simulink model "ClimaRateLimMgr"  (R2022b)
settings: Solver=FixedStepDiscrete  StartTime=0.0  StopTime=10.0  FixedStep=0.2  RelTol=1e-3
(part 2 of 2)

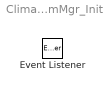
== system ClimaRateLimMgr/ClimaRateLimMgr_Init ==
  Event Listener : EventListener  EventName=reset

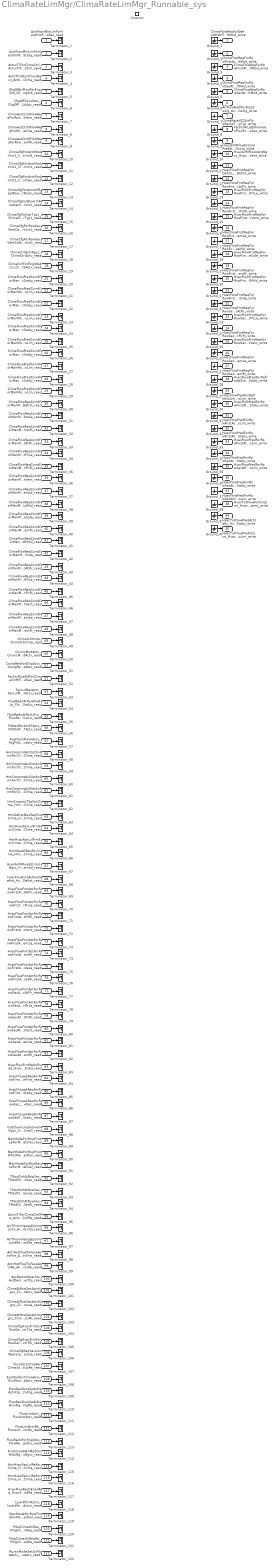
== system ClimaRateLimMgr/ClimaRateLimMgr_Runnable_sys ==
  ActrPosnMinLimForVentForRowSec_ActrPosnMinLimForVentForRowSec_read : Inport
  ActrPosnMinLimForVentForRowSecPredForStaby_ActrPosnMinLimForVentForRowSecPredForStaby_read : Inport  Port=2
  ActvnTiForClimaCtrl_ActvnTiForClimaCtrl_read : Inport  Port=3
  AmbTFildForClimaNgcc_AmbTFildForClima_read : Inport  Port=4
  ChgOfAirFlowForEngStb_ChgOfAirFlowForEngStb_read : Inport  Port=5
  ChgOfFanLvlAut_ChgOfFanLvlAut_read : Inport  Port=6
  ClimaAutCtrldFlowReqForRowSecAtVent_ClimaAutCtrldFlowReqForRowSecAtVent_read : Inport  Port=7
  ClimaAutCtrldFlowReqForRowSecAtVent_ClimaAutCtrldFlowReqForRowSecAtVentLe_read : Inport  Port=8
  ClimaAutCtrldFlowReqForRowSecAtVent_ClimaAutCtrldFlowReqForRowSecAtVentRi_read : Inport  Port=9
  ClimaCfgForActrModVrnt1_ClimaCfgForActrInd1_read : Inport  Port=10
  ClimaCfgForActrModVrnt1_ClimaCfgForActrModVrnt1_read : Inport  Port=11
  ClimaCfgForActrModVrnt1_ClimaCfgForActrModVrntCen_read : Inport  Port=12
  ClimaCfgForAmntOfSeatRow1_ClimaCfgForAmntOfSeatRow1_read : Inport  Port=13
  ClimaCfgForBlowrInRowSecVentSidePrsnt1_ClimaCfgForBlowrInRowSecVentSidePrsnt1_read : Inport  Port=14
  ClimaCfgForCarTyp1_ClimaCfgForCarTyp1_read : Inport  Port=15
  ClimaCfgForRowSecVentCentrVrnt1_ClimaCfgForRowSecVentCentrVrnt1_read : Inport  Port=16
  ClimaCfgForRowSecVentSidePrsnt1_ClimaCfgForRowSecVentSidePrsnt1_read : Inport  Port=17
  ClimaCtrlActvNgcc_ClimaCtrlActv_read : Inport  Port=18
  ClimaCtrlForEngStbActv_ClimaCtrlForEngStbActv_read : Inport  Port=19
  ClimaFlowPredCorrdForManModForDefrstAtStaby_ClimaFlowPredCorrdForManModForDefrstAtStaby_read : Inport  Port=20
  ClimaFlowPredCorrdForManModForDefrstNoLim_ClimaFlowPredCorrdForManModForDefrstNoLim_read : Inport  Port=21
  ClimaFlowPredCorrdForManModForRowFirstAtFlrAtStaby_ClimaFlowPredCorrdForManModForRowFirstAtFlrAtStaby_read : Inport  Port=22
  ClimaFlowPredCorrdForManModForRowFirstAtFlrNoLim_ClimaFlowPredCorrdForManModForRowFirstAtFlrNoLim_read : Inport  Port=23
  ClimaFlowPredCorrdForManModForRowFirstAtVentAtStaby_ClimaFlowPredCorrdForManModForRowFirstAtVentAtStaby_read : Inport  Port=24
  ClimaFlowPredCorrdForManModForRowFirstAtVentNoLim_ClimaFlowPredCorrdForManModForRowFirstAtVentNoLim_read : Inport  Port=25
  ClimaFlowPredCorrdForManModForRowSecAtFlrAtStaby_ClimaFlowPredCorrdForManModForRowSecAtFlrAtStaby_read : Inport  Port=26
  ClimaFlowPredCorrdForManModForRowSecAtFlrNoLim_ClimaFlowPredCorrdForManModForRowSecAtFlrNoLim_read : Inport  Port=27
  ClimaFlowPredCorrdForManModForRowSecAtVentAtStaby_ClimaFlowPredCorrdForManModForRowSecAtVentAtStaby_read : Inport  Port=28
  ClimaFlowPredCorrdForManModForRowSecAtVentNoLim_ClimaFlowPredCorrdForManModForRowSecAtVentNoLim_read : Inport  Port=29
  ClimaFlowReqCorrdForManModForDefrst_ClimaFlowReqCorrdForManModForDefrst_read : Inport  Port=30
  ClimaFlowReqCorrdForManModForDefrst_ClimaFlowReqCorrdForManModForDefrstLe_read : Inport  Port=31
  ClimaFlowReqCorrdForManModForDefrst_ClimaFlowReqCorrdForManModForDefrstRi_read : Inport  Port=32
  ClimaFlowReqCorrdForManModForRowFirstAtFlr_ClimaFlowReqCorrdForManModForRowFirstAtFlr_read : Inport  Port=33
  ClimaFlowReqCorrdForManModForRowFirstAtFlr_ClimaFlowReqCorrdForManModForRowFirstAtFlrLe_read : Inport  Port=34
  ClimaFlowReqCorrdForManModForRowFirstAtFlr_ClimaFlowReqCorrdForManModForRowFirstAtFlrRi_read : Inport  Port=35
  ClimaFlowReqCorrdForManModForRowFirstAtVent_ClimaFlowReqCorrdForManModForRowFirstAtVent_read : Inport  Port=36
  ClimaFlowReqCorrdForManModForRowFirstAtVent_ClimaFlowReqCorrdForManModForRowFirstAtVentLe_read : Inport  Port=37
  ClimaFlowReqCorrdForManModForRowFirstAtVent_ClimaFlowReqCorrdForManModForRowFirstAtVentLeMid_read : Inport  Port=38
  ClimaFlowReqCorrdForManModForRowFirstAtVent_ClimaFlowReqCorrdForManModForRowFirstAtVentLeSide_read : Inport  Port=39
  ClimaFlowReqCorrdForManModForRowFirstAtVent_ClimaFlowReqCorrdForManModForRowFirstAtVentRi_read : Inport  Port=40
  ClimaFlowReqCorrdForManModForRowFirstAtVent_ClimaFlowReqCorrdForManModForRowFirstAtVentRiMid_read : Inport  Port=41
  ClimaFlowReqCorrdForManModForRowFirstAtVent_ClimaFlowReqCorrdForManModForRowFirstAtVentRiSide_read : Inport  Port=42
  ClimaFlowReqCorrdForManModForRowSecAtFlr_ClimaFlowReqCorrdForManModForRowSecAtFlr_read : Inport  Port=43
  ClimaFlowReqCorrdForManModForRowSecAtFlr_ClimaFlowReqCorrdForManModForRowSecAtFlrLe_read : Inport  Port=44
  ClimaFlowReqCorrdForManModForRowSecAtFlr_ClimaFlowReqCorrdForManModForRowSecAtFlrRi_read : Inport  Port=45
  ClimaFlowReqCorrdForManModForRowSecAtVent_ClimaFlowReqCorrdForManModForRowSecAtVent_read : Inport  Port=46
  ClimaFlowReqCorrdForManModForRowSecAtVent_ClimaFlowReqCorrdForManModForRowSecAtVentLe_read : Inport  Port=47
  ClimaFlowReqCorrdForManModForRowSecAtVent_ClimaFlowReqCorrdForManModForRowSecAtVentRi_read : Inport  Port=48
  ClimaStStrtUp_ClimaStStrtUp_read : Inport  Port=49
  CnvincModActv_CnvincModActv_read : Inport  Port=50
  CoolgReqForDispActv_CoolgReqForDispActv_read : Inport  Port=51
  FacForRiLeDifForClimaCmftFlowForRowSec_FacForRiLeDifForClimaCmftFlowForRowSec_read : Inport  Port=52
  FanLvlMaxActv_FanLvlMaxActv_read : Inport  Port=53
  FlowRednAtPwrAllwdLo_FlowRednAtPwrAllwdLo_read : Inport  Port=54
  FlowRednAtPwrLvlLo_FlowRednAtPwrLvlLo_read : Inport  Port=55
  FltModForAmbTActv_FltModForAmbTActv_read : Inport  Port=56
  FogFlashPrevnActv_FogFlashPrevnActv_read : Inport  Port=57
  HmiCmptmtAirDistbnFrntForClima_HmiCmptmtAirDistbnFrntForClima_read : Inport  Port=58
  HmiCmptmtAirDistbnFrntForClima_HmiCmptmtAirDistbnFrntRiForClima_read : Inport  Port=59
  HmiCmptmtAirDistbnFrntForClima_HmiCmptmtAirDistbnReForClima_read : Inport  Port=60
  HmiCmptmtAirDistbnFrntForClima_HmiCmptmtAirDistbnReRiForClima_read : Inport  Port=61
  HmiCmptmtTSpForClima_HmiCmptmtTSpForClima_read : Inport  Port=62
  HmiDefrstMaxReqForClima_HmiDefrstMaxReqForClima_read : Inport  Port=63
  HmiHvacFanLvlFrntForClima_HmiHvacFanLvlFrntForClima_read : Inport  Port=64
  HmiHvacFanLvlFrntForClima_HmiHvacFanLvlFrntRiForClima_read : Inport  Port=65
  HmiMaxACReqForClima_HmiMaxACReqForClima_read : Inport  Port=66
  HvacAirMFlowEstimd_Ngcc_HvacAirMFlowEstimd_read : Inport  Port=67
  HvacFlowFrntActForDefrst_HvacFlowFrntActForDefrst_read : Inport  Port=68
  HvacFlowFrntActForRowFirstAtFlr_HvacFlowFrntActForRowFirstAtFlr_read : Inport  Port=69
  HvacFlowFrntActForRowFirstAtFlr_HvacFlowFrntActForRowFirstAtFlrLe_read : Inport  Port=70
  HvacFlowFrntActForRowFirstAtFlr_HvacFlowFrntActForRowFirstAtFlrRi_read : Inport  Port=71
  HvacFlowFrntActForRowFirstAtVent_HvacFlowFrntActForRowFirstAtVent_read : Inport  Port=72
  HvacFlowFrntActForRowFirstAtVent_HvacFlowFrntActForRowFirstAtVentLe_read : Inport  Port=73
  HvacFlowFrntActForRowFirstAtVent_HvacFlowFrntActForRowFirstAtVentRi_read : Inport  Port=74
  HvacFlowFrntActForRowFirstAtVent_HvacFlowFrntActForRowFirstAtVentSideLe_read : Inport  Port=75
  HvacFlowFrntActForRowFirstAtVent_HvacFlowFrntActForRowFirstAtVentSideRi_read : Inport  Port=76
  HvacFlowFrntActForRowSecAtFlr_HvacFlowFrntActForRowSecAtFlr_read : Inport  Port=77
  HvacFlowFrntActForRowSecAtFlr_HvacFlowFrntActForRowSecAtFlrLe_read : Inport  Port=78
  HvacFlowFrntActForRowSecAtFlr_HvacFlowFrntActForRowSecAtFlrRi_read : Inport  Port=79
  HvacFlowFrntActForRowSecAtVent_HvacFlowFrntActForRowSecAtVent_read : Inport  Port=80
  HvacFlowFrntActForRowSecAtVent_HvacFlowFrntActForRowSecAtVentLe_read : Inport  Port=81
  HvacFlowFrntActForRowSecAtVent_HvacFlowFrntActForRowSecAtVentRi_read : Inport  Port=82
  HvacFlowFrntRednEnad_HvacFlowFrntRednEnad_read : Inport  Port=83
  HvacFlrLeakReqForRowFirst_HvacFlrLeakReqForRowFirst_read : Inport  Port=84
  HvacFlrLeakReqForRowFirstPredForStaby_HvacFlrLeakReqForRowFirstPredForStaby_read : Inport  Port=85
  HvacFlrLeakReqForRowSec_HvacFlrLeakReqForRowSec_read : Inport  Port=86
  HvacFlrLeakReqForRowSecPredForStaby_HvacFlrLeakReqForRowSecPredForStaby_read : Inport  Port=87
  InteCleanUnpleSmell_Ngcc_InteCleanUnpleSmell_read : Inport  Port=88
  ManModeForRowFirstLeForModelDial_ManModeForRowFirstLeForModelDial_read : Inport  Port=89
  ManModeForRowFirstRiForModelDial_ManModeForRowFirstRiForModelDial_read : Inport  Port=90
  ManModeForRowSecLeForModelDial_ManModeForRowSecLeForModelDial_read : Inport  Port=91
  TModCtrlAtRowSec_TModCtrlAtRowSec_read : Inport  Port=92
  TModCtrlAtRowSec_TModCtrlAtRowSecLe_read : Inport  Port=93
  TModCtrlAtRowSec_TModCtrlAtRowSecRi_read : Inport  Port=94
  ActvnTiForClimaCtrlRe_ActvnTiForClimaCtrlRe_read : Inport  Port=95
  AirTFromHeatgEstimdLimd_AirTFromHeatgEstimdLimd_read : Inport  Port=96
  AirTFromHeatgEstimdLimdRe_AirTFromHeatgEstimdLimdRe_read : Inport  Port=97
  AntiHotFlowToFaceActvFrnt_AntiHotFlowToFaceActvFrnt_read : Inport  Port=98
  AntiHotFlowToFaceActvRe_AntiHotFlowToFaceActvRe_read : Inport  Port=99
  AutDemstOperSts_AutDemstOperSts_read : Inport  Port=100
  ClimaAtRowSecActvNgcc_ClimaAtRowSecActv_read : Inport  Port=101
  ClimaAtRowSecActvNgcc_ClimaAtRowSecActvLe_read : Inport  Port=102
  ClimaAtRowSecActvNgcc_ClimaAtRowSecActvRi_read : Inport  Port=103
  ClimaCfgHvacFrntForRowSecForActrTLe_ClimaCfgHvacFrntForRowSecForActrTLe_read : Inport  Port=104
  ClimaCfgHvacFrntForRowSecForActrTRi_ClimaCfgHvacFrntForRowSecForActrTRi_read : Inport  Port=105
  ClimaCfgReqVersionReplace_ClimaCfgReqVersionReplace_read : Inport  Port=106
  ClimaStStrtUpRe_ClimaStStrtUpRe_read : Inport  Port=107
  EcoModForClimaActv_EcoModForClimaActv_read : Inport  Port=108
  FlowDecDurgSpdIdleActvFlg_FlowDecDurgSpdIdleActvFlg_read : Inport  Port=109
  FlowDecDurgSpdIdleActvFlgRe_FlowDecDurgSpdIdleActvFlgRe_read : Inport  Port=110
  FlowLimActv_FlowLimActv_read : Inport  Port=111
  FlowLimActvRe_FlowLimActvRe_read : Inport  Port=112
  FlowRednForEngStby_FlowRednForEngStby_read : Inport  Port=113
  FrntClimaManModCmftStsNgcc_FrntClimaManModCmftStsNgcc_read : Inport  Port=114
  HmiHvacFanLvlReForClima_HmiHvacFanLvlReForClima_read : Inport  Port=115
  HmiHvacFanLvlReForClima_HmiHvacFanLvlReRiForClima_read : Inport  Port=116
  HvacFlowRednEnadRe_HvacFlowRednEnadRe_read : Inport  Port=117
  LowrWtrTActvn_LowrWtrTActvn_read : Inport  Port=118
  ManModeForRowThrdForModelDial_ManModeForRowThrdForModelDial_read : Inport  Port=119
  PrkgClimaOnReq_PrkgClimaOnReq_read : Inport  Port=120
  PrkgClimaOnReqRe_PrkgClimaOnReqRe_read : Inport  Port=121
  RuranModeSetAirFlowActv_RuranModeSetAirFlowActv_read : Inport  Port=122
  function : TriggerPort  TriggerType=function-call  VariantControl=(inherit)  SampleTimeType=periodic  SampleTime=-1
  Ground_{1..40} (40 alike) : Ground
  Terminator_{1..122} (122 alike) : Terminator
  ClimaFlowReqForDefrstWithFltMod_ClimaFlowReqForDefrstWithFltMod_write : Outport
      settings: EnsureOutportIsVirtual=on
  ClimaFlowReqForRowFirstAtFlrWithFltMod_ClimaFlowReqForRowFirstAtFlrWithFltMod_write : Outport  Port=2
      settings: EnsureOutportIsVirtual=on
  ClimaFlowReqForRowFirstAtVentWithFltMod_ClimaFlowReqForRowFirstAtVentWithFltMod_write : Outport  Port=3
      settings: EnsureOutportIsVirtual=on
  ClimaFlowReqForRowSecAtFlrWithFltMod_ClimaFlowReqForRowSecAtFlrWithFltMod_write : Outport  Port=4
      settings: EnsureOutportIsVirtual=on
  ClimaFlowReqForRowSecAtVentWithFltMod_ClimaFlowReqForRowSecAtVentWithFltMod_write : Outport  Port=5
      settings: EnsureOutportIsVirtual=on
  AirFlowAddlForDispCoolg_AirFlowAddlForDispCoolg_write : Outport  Port=6
      settings: EnsureOutportIsVirtual=on
  ClimaRateVCCAirFlowRampTyp_ClimaRateVCCAirFlowRampTyp_write : Outport  Port=7
      settings: EnsureOutportIsVirtual=on
  FacForRiLeDifForHvacFlowForVentRowSec_FacForRiLeDifForHvacFlowForVentRowSec_write : Outport  Port=8
      settings: EnsureOutportIsVirtual=on
  HvacAirMFlowEstimdPredAtStabyForCoolg_HvacAirMFlowEstimdPredAtStabyForCoolg_write : Outport  Port=9
      settings: EnsureOutportIsVirtual=on
  HvacAirMFlowVentNgcc_HvacAirMFlowVent_write : Outport  Port=10
      settings: EnsureOutportIsVirtual=on
  HvacFlowFrntReqForDefrst_HvacFlowFrntReqForDefrst_write : Outport  Port=11
      settings: EnsureOutportIsVirtual=on
  HvacFlowFrntReqForRowFirstAtFlr_HvacFlowFrntReqForRowFirstAtFlr_write : Outport  Port=12
      settings: EnsureOutportIsVirtual=on
  HvacFlowFrntReqForRowFirstAtFlr_HvacFlowFrntReqForRowFirstAtFlrLe_write : Outport  Port=13
      settings: EnsureOutportIsVirtual=on
  HvacFlowFrntReqForRowFirstAtFlr_HvacFlowFrntReqForRowFirstAtFlrRi_write : Outport  Port=14
      settings: EnsureOutportIsVirtual=on
  HvacFlowFrntReqForRowFirstAtVent_HvacFlowFrntReqForRowFirstAtVent_write : Outport  Port=15
      settings: EnsureOutportIsVirtual=on
  HvacFlowFrntReqForRowFirstAtVent_HvacFlowFrntReqForRowFirstAtVentLe_write : Outport  Port=16
      settings: EnsureOutportIsVirtual=on
  HvacFlowFrntReqForRowFirstAtVent_HvacFlowFrntReqForRowFirstAtVentLeMid_write : Outport  Port=17
      settings: EnsureOutportIsVirtual=on
  HvacFlowFrntReqForRowFirstAtVent_HvacFlowFrntReqForRowFirstAtVentLeSide_write : Outport  Port=18
      settings: EnsureOutportIsVirtual=on
  HvacFlowFrntReqForRowFirstAtVent_HvacFlowFrntReqForRowFirstAtVentRi_write : Outport  Port=19
      settings: EnsureOutportIsVirtual=on
  HvacFlowFrntReqForRowFirstAtVent_HvacFlowFrntReqForRowFirstAtVentRiMid_write : Outport  Port=20
      settings: EnsureOutportIsVirtual=on
  HvacFlowFrntReqForRowFirstAtVent_HvacFlowFrntReqForRowFirstAtVentRiSide_write : Outport  Port=21
      settings: EnsureOutportIsVirtual=on
  HvacFlowFrntReqForRowSecAtFlr_HvacFlowFrntReqForRowSecAtFlr_write : Outport  Port=22
      settings: EnsureOutportIsVirtual=on
  HvacFlowFrntReqForRowSecAtFlr_HvacFlowFrntReqForRowSecAtFlrLe_write : Outport  Port=23
      settings: EnsureOutportIsVirtual=on
  HvacFlowFrntReqForRowSecAtFlr_HvacFlowFrntReqForRowSecAtFlrRi_write : Outport  Port=24
      settings: EnsureOutportIsVirtual=on
  HvacFlowFrntReqForRowSecAtVent_HvacFlowFrntReqForRowSecAtVent_write : Outport  Port=25
      settings: EnsureOutportIsVirtual=on
  HvacFlowFrntReqForRowSecAtVent_HvacFlowFrntReqForRowSecAtVentLe_write : Outport  Port=26
      settings: EnsureOutportIsVirtual=on
  HvacFlowFrntReqForRowSecAtVent_HvacFlowFrntReqForRowSecAtVentRi_write : Outport  Port=27
      settings: EnsureOutportIsVirtual=on
  HvacFlowPredForDefrstAtStaby_HvacFlowPredForDefrstAtStaby_write : Outport  Port=28
      settings: EnsureOutportIsVirtual=on
  HvacFlowPredForDefrstNoLim_HvacFlowPredForDefrstNoLim_write : Outport  Port=29
      settings: EnsureOutportIsVirtual=on
  HvacFlowPredForRowFirstAtFlrAtStaby_HvacFlowPredForRowFirstAtFlrAtStaby_write : Outport  Port=30
      settings: EnsureOutportIsVirtual=on
  HvacFlowPredForRowFirstAtFlrNoLim_HvacFlowPredForRowFirstAtFlrNoLim_write : Outport  Port=31
      settings: EnsureOutportIsVirtual=on
  HvacFlowPredForRowFirstAtVentAtStaby_HvacFlowPredForRowFirstAtVentAtStaby_write : Outport  Port=32
      settings: EnsureOutportIsVirtual=on
  HvacFlowPredForRowFirstAtVentNoLim_HvacFlowPredForRowFirstAtVentNoLim_write : Outport  Port=33
      settings: EnsureOutportIsVirtual=on
  HvacFlowPredForRowSecAtFlrAtStaby_HvacFlowPredForRowSecAtFlrAtStaby_write : Outport  Port=34
      settings: EnsureOutportIsVirtual=on
  HvacFlowPredForRowSecAtFlrNoLim_HvacFlowPredForRowSecAtFlrNoLim_write : Outport  Port=35
      settings: EnsureOutportIsVirtual=on
  HvacFlowPredForRowSecAtVentAtStaby_HvacFlowPredForRowSecAtVentAtStaby_write : Outport  Port=36
      settings: EnsureOutportIsVirtual=on
  HvacFlowPredForRowSecAtVentNoLim_HvacFlowPredForRowSecAtVentNoLim_write : Outport  Port=37
      settings: EnsureOutportIsVirtual=on
  HvacTotFlowForCmptmt_HvacTotFlowForCmptmt_write : Outport  Port=38
      settings: EnsureOutportIsVirtual=on
  HvacTotFlowPredAtStaby_HvacTotFlowPredAtStaby_write : Outport  Port=39
      settings: EnsureOutportIsVirtual=on
  HvacTotFlowPredNoLim_HvacTotFlowPredNoLim_write : Outport  Port=40
      settings: EnsureOutportIsVirtual=on
  wires:
  ActrPosnMinLimForVentForRowSec_ActrPosnMinLimForVentForRowSec_read/1 -> Terminator_1/1
  ActrPosnMinLimForVentForRowSecPredForStaby_ActrPosnMinLimForVentForRowSecPredForStaby_read/1 -> Terminator_2/1
  ActvnTiForClimaCtrl_ActvnTiForClimaCtrl_read/1 -> Terminator_3/1
  AmbTFildForClimaNgcc_AmbTFildForClima_read/1 -> Terminator_4/1
  ChgOfAirFlowForEngStb_ChgOfAirFlowForEngStb_read/1 -> Terminator_5/1
  ChgOfFanLvlAut_ChgOfFanLvlAut_read/1 -> Terminator_6/1
  ClimaAutCtrldFlowReqForRowSecAtVent_ClimaAutCtrldFlowReqForRowSecAtVent_read/1 -> Terminator_7/1
  ClimaAutCtrldFlowReqForRowSecAtVent_ClimaAutCtrldFlowReqForRowSecAtVentLe_read/1 -> Terminator_8/1
  ClimaAutCtrldFlowReqForRowSecAtVent_ClimaAutCtrldFlowReqForRowSecAtVentRi_read/1 -> Terminator_9/1
  ClimaCfgForActrModVrnt1_ClimaCfgForActrInd1_read/1 -> Terminator_10/1
  ClimaCfgForActrModVrnt1_ClimaCfgForActrModVrnt1_read/1 -> Terminator_11/1
  ClimaCfgForActrModVrnt1_ClimaCfgForActrModVrntCen_read/1 -> Terminator_12/1
  ClimaCfgForAmntOfSeatRow1_ClimaCfgForAmntOfSeatRow1_read/1 -> Terminator_13/1
  ClimaCfgForBlowrInRowSecVentSidePrsnt1_ClimaCfgForBlowrInRowSecVentSidePrsnt1_read/1 -> Terminator_14/1
  ClimaCfgForCarTyp1_ClimaCfgForCarTyp1_read/1 -> Terminator_15/1
  ClimaCfgForRowSecVentCentrVrnt1_ClimaCfgForRowSecVentCentrVrnt1_read/1 -> Terminator_16/1
  ClimaCfgForRowSecVentSidePrsnt1_ClimaCfgForRowSecVentSidePrsnt1_read/1 -> Terminator_17/1
  ClimaCtrlActvNgcc_ClimaCtrlActv_read/1 -> Terminator_18/1
  ClimaCtrlForEngStbActv_ClimaCtrlForEngStbActv_read/1 -> Terminator_19/1
  ClimaFlowPredCorrdForManModForDefrstAtStaby_ClimaFlowPredCorrdForManModForDefrstAtStaby_read/1 -> Terminator_20/1
  ClimaFlowPredCorrdForManModForDefrstNoLim_ClimaFlowPredCorrdForManModForDefrstNoLim_read/1 -> Terminator_21/1
  ClimaFlowPredCorrdForManModForRowFirstAtFlrAtStaby_ClimaFlowPredCorrdForManModForRowFirstAtFlrAtStaby_read/1 -> Terminator_22/1
  ClimaFlowPredCorrdForManModForRowFirstAtFlrNoLim_ClimaFlowPredCorrdForManModForRowFirstAtFlrNoLim_read/1 -> Terminator_23/1
  ClimaFlowPredCorrdForManModForRowFirstAtVentAtStaby_ClimaFlowPredCorrdForManModForRowFirstAtVentAtStaby_read/1 -> Terminator_24/1
  ClimaFlowPredCorrdForManModForRowFirstAtVentNoLim_ClimaFlowPredCorrdForManModForRowFirstAtVentNoLim_read/1 -> Terminator_25/1
  ClimaFlowPredCorrdForManModForRowSecAtFlrAtStaby_ClimaFlowPredCorrdForManModForRowSecAtFlrAtStaby_read/1 -> Terminator_26/1
  ClimaFlowPredCorrdForManModForRowSecAtFlrNoLim_ClimaFlowPredCorrdForManModForRowSecAtFlrNoLim_read/1 -> Terminator_27/1
  ClimaFlowPredCorrdForManModForRowSecAtVentAtStaby_ClimaFlowPredCorrdForManModForRowSecAtVentAtStaby_read/1 -> Terminator_28/1
  ClimaFlowPredCorrdForManModForRowSecAtVentNoLim_ClimaFlowPredCorrdForManModForRowSecAtVentNoLim_read/1 -> Terminator_29/1
  ClimaFlowReqCorrdForManModForDefrst_ClimaFlowReqCorrdForManModForDefrst_read/1 -> Terminator_30/1
  ClimaFlowReqCorrdForManModForDefrst_ClimaFlowReqCorrdForManModForDefrstLe_read/1 -> Terminator_31/1
  ClimaFlowReqCorrdForManModForDefrst_ClimaFlowReqCorrdForManModForDefrstRi_read/1 -> Terminator_32/1
  ClimaFlowReqCorrdForManModForRowFirstAtFlr_ClimaFlowReqCorrdForManModForRowFirstAtFlr_read/1 -> Terminator_33/1
  ClimaFlowReqCorrdForManModForRowFirstAtFlr_ClimaFlowReqCorrdForManModForRowFirstAtFlrLe_read/1 -> Terminator_34/1
  ClimaFlowReqCorrdForManModForRowFirstAtFlr_ClimaFlowReqCorrdForManModForRowFirstAtFlrRi_read/1 -> Terminator_35/1
  ClimaFlowReqCorrdForManModForRowFirstAtVent_ClimaFlowReqCorrdForManModForRowFirstAtVent_read/1 -> Terminator_36/1
  ClimaFlowReqCorrdForManModForRowFirstAtVent_ClimaFlowReqCorrdForManModForRowFirstAtVentLe_read/1 -> Terminator_37/1
  ClimaFlowReqCorrdForManModForRowFirstAtVent_ClimaFlowReqCorrdForManModForRowFirstAtVentLeMid_read/1 -> Terminator_38/1
  ClimaFlowReqCorrdForManModForRowFirstAtVent_ClimaFlowReqCorrdForManModForRowFirstAtVentLeSide_read/1 -> Terminator_39/1
  ClimaFlowReqCorrdForManModForRowFirstAtVent_ClimaFlowReqCorrdForManModForRowFirstAtVentRi_read/1 -> Terminator_40/1
  ClimaFlowReqCorrdForManModForRowFirstAtVent_ClimaFlowReqCorrdForManModForRowFirstAtVentRiMid_read/1 -> Terminator_41/1
  ClimaFlowReqCorrdForManModForRowFirstAtVent_ClimaFlowReqCorrdForManModForRowFirstAtVentRiSide_read/1 -> Terminator_42/1
  ClimaFlowReqCorrdForManModForRowSecAtFlr_ClimaFlowReqCorrdForManModForRowSecAtFlr_read/1 -> Terminator_43/1
  ClimaFlowReqCorrdForManModForRowSecAtFlr_ClimaFlowReqCorrdForManModForRowSecAtFlrLe_read/1 -> Terminator_44/1
  ClimaFlowReqCorrdForManModForRowSecAtFlr_ClimaFlowReqCorrdForManModForRowSecAtFlrRi_read/1 -> Terminator_45/1
  ClimaFlowReqCorrdForManModForRowSecAtVent_ClimaFlowReqCorrdForManModForRowSecAtVent_read/1 -> Terminator_46/1
  ClimaFlowReqCorrdForManModForRowSecAtVent_ClimaFlowReqCorrdForManModForRowSecAtVentLe_read/1 -> Terminator_47/1
  ClimaFlowReqCorrdForManModForRowSecAtVent_ClimaFlowReqCorrdForManModForRowSecAtVentRi_read/1 -> Terminator_48/1
  ClimaStStrtUp_ClimaStStrtUp_read/1 -> Terminator_49/1
  CnvincModActv_CnvincModActv_read/1 -> Terminator_50/1
  CoolgReqForDispActv_CoolgReqForDispActv_read/1 -> Terminator_51/1
  FacForRiLeDifForClimaCmftFlowForRowSec_FacForRiLeDifForClimaCmftFlowForRowSec_read/1 -> Terminator_52/1
  FanLvlMaxActv_FanLvlMaxActv_read/1 -> Terminator_53/1
  FlowRednAtPwrAllwdLo_FlowRednAtPwrAllwdLo_read/1 -> Terminator_54/1
  FlowRednAtPwrLvlLo_FlowRednAtPwrLvlLo_read/1 -> Terminator_55/1
  FltModForAmbTActv_FltModForAmbTActv_read/1 -> Terminator_56/1
  FogFlashPrevnActv_FogFlashPrevnActv_read/1 -> Terminator_57/1
  HmiCmptmtAirDistbnFrntForClima_HmiCmptmtAirDistbnFrntForClima_read/1 -> Terminator_58/1
  HmiCmptmtAirDistbnFrntForClima_HmiCmptmtAirDistbnFrntRiForClima_read/1 -> Terminator_59/1
  HmiCmptmtAirDistbnFrntForClima_HmiCmptmtAirDistbnReForClima_read/1 -> Terminator_60/1
  HmiCmptmtAirDistbnFrntForClima_HmiCmptmtAirDistbnReRiForClima_read/1 -> Terminator_61/1
  HmiCmptmtTSpForClima_HmiCmptmtTSpForClima_read/1 -> Terminator_62/1
  HmiDefrstMaxReqForClima_HmiDefrstMaxReqForClima_read/1 -> Terminator_63/1
  HmiHvacFanLvlFrntForClima_HmiHvacFanLvlFrntForClima_read/1 -> Terminator_64/1
  HmiHvacFanLvlFrntForClima_HmiHvacFanLvlFrntRiForClima_read/1 -> Terminator_65/1
  HmiMaxACReqForClima_HmiMaxACReqForClima_read/1 -> Terminator_66/1
  HvacAirMFlowEstimd_Ngcc_HvacAirMFlowEstimd_read/1 -> Terminator_67/1
  HvacFlowFrntActForDefrst_HvacFlowFrntActForDefrst_read/1 -> Terminator_68/1
  HvacFlowFrntActForRowFirstAtFlr_HvacFlowFrntActForRowFirstAtFlr_read/1 -> Terminator_69/1
  HvacFlowFrntActForRowFirstAtFlr_HvacFlowFrntActForRowFirstAtFlrLe_read/1 -> Terminator_70/1
  HvacFlowFrntActForRowFirstAtFlr_HvacFlowFrntActForRowFirstAtFlrRi_read/1 -> Terminator_71/1
  HvacFlowFrntActForRowFirstAtVent_HvacFlowFrntActForRowFirstAtVent_read/1 -> Terminator_72/1
  HvacFlowFrntActForRowFirstAtVent_HvacFlowFrntActForRowFirstAtVentLe_read/1 -> Terminator_73/1
  HvacFlowFrntActForRowFirstAtVent_HvacFlowFrntActForRowFirstAtVentRi_read/1 -> Terminator_74/1
  HvacFlowFrntActForRowFirstAtVent_HvacFlowFrntActForRowFirstAtVentSideLe_read/1 -> Terminator_75/1
  HvacFlowFrntActForRowFirstAtVent_HvacFlowFrntActForRowFirstAtVentSideRi_read/1 -> Terminator_76/1
  HvacFlowFrntActForRowSecAtFlr_HvacFlowFrntActForRowSecAtFlr_read/1 -> Terminator_77/1
  HvacFlowFrntActForRowSecAtFlr_HvacFlowFrntActForRowSecAtFlrLe_read/1 -> Terminator_78/1
  HvacFlowFrntActForRowSecAtFlr_HvacFlowFrntActForRowSecAtFlrRi_read/1 -> Terminator_79/1
  HvacFlowFrntActForRowSecAtVent_HvacFlowFrntActForRowSecAtVent_read/1 -> Terminator_80/1
  HvacFlowFrntActForRowSecAtVent_HvacFlowFrntActForRowSecAtVentLe_read/1 -> Terminator_81/1
  HvacFlowFrntActForRowSecAtVent_HvacFlowFrntActForRowSecAtVentRi_read/1 -> Terminator_82/1
  HvacFlowFrntRednEnad_HvacFlowFrntRednEnad_read/1 -> Terminator_83/1
  HvacFlrLeakReqForRowFirst_HvacFlrLeakReqForRowFirst_read/1 -> Terminator_84/1
  HvacFlrLeakReqForRowFirstPredForStaby_HvacFlrLeakReqForRowFirstPredForStaby_read/1 -> Terminator_85/1
  HvacFlrLeakReqForRowSec_HvacFlrLeakReqForRowSec_read/1 -> Terminator_86/1
  HvacFlrLeakReqForRowSecPredForStaby_HvacFlrLeakReqForRowSecPredForStaby_read/1 -> Terminator_87/1
  InteCleanUnpleSmell_Ngcc_InteCleanUnpleSmell_read/1 -> Terminator_88/1
  ManModeForRowFirstLeForModelDial_ManModeForRowFirstLeForModelDial_read/1 -> Terminator_89/1
  ManModeForRowFirstRiForModelDial_ManModeForRowFirstRiForModelDial_read/1 -> Terminator_90/1
  ManModeForRowSecLeForModelDial_ManModeForRowSecLeForModelDial_read/1 -> Terminator_91/1
  TModCtrlAtRowSec_TModCtrlAtRowSec_read/1 -> Terminator_92/1
  TModCtrlAtRowSec_TModCtrlAtRowSecLe_read/1 -> Terminator_93/1
  TModCtrlAtRowSec_TModCtrlAtRowSecRi_read/1 -> Terminator_94/1
  ActvnTiForClimaCtrlRe_ActvnTiForClimaCtrlRe_read/1 -> Terminator_95/1
  AirTFromHeatgEstimdLimd_AirTFromHeatgEstimdLimd_read/1 -> Terminator_96/1
  AirTFromHeatgEstimdLimdRe_AirTFromHeatgEstimdLimdRe_read/1 -> Terminator_97/1
  AntiHotFlowToFaceActvFrnt_AntiHotFlowToFaceActvFrnt_read/1 -> Terminator_98/1
  AntiHotFlowToFaceActvRe_AntiHotFlowToFaceActvRe_read/1 -> Terminator_99/1
  AutDemstOperSts_AutDemstOperSts_read/1 -> Terminator_100/1
  ClimaAtRowSecActvNgcc_ClimaAtRowSecActv_read/1 -> Terminator_101/1
  ClimaAtRowSecActvNgcc_ClimaAtRowSecActvLe_read/1 -> Terminator_102/1
  ClimaAtRowSecActvNgcc_ClimaAtRowSecActvRi_read/1 -> Terminator_103/1
  ClimaCfgHvacFrntForRowSecForActrTLe_ClimaCfgHvacFrntForRowSecForActrTLe_read/1 -> Terminator_104/1
  ClimaCfgHvacFrntForRowSecForActrTRi_ClimaCfgHvacFrntForRowSecForActrTRi_read/1 -> Terminator_105/1
  ClimaCfgReqVersionReplace_ClimaCfgReqVersionReplace_read/1 -> Terminator_106/1
  ClimaStStrtUpRe_ClimaStStrtUpRe_read/1 -> Terminator_107/1
  EcoModForClimaActv_EcoModForClimaActv_read/1 -> Terminator_108/1
  FlowDecDurgSpdIdleActvFlg_FlowDecDurgSpdIdleActvFlg_read/1 -> Terminator_109/1
  FlowDecDurgSpdIdleActvFlgRe_FlowDecDurgSpdIdleActvFlgRe_read/1 -> Terminator_110/1
  FlowLimActv_FlowLimActv_read/1 -> Terminator_111/1
  FlowLimActvRe_FlowLimActvRe_read/1 -> Terminator_112/1
  FlowRednForEngStby_FlowRednForEngStby_read/1 -> Terminator_113/1
  FrntClimaManModCmftStsNgcc_FrntClimaManModCmftStsNgcc_read/1 -> Terminator_114/1
  HmiHvacFanLvlReForClima_HmiHvacFanLvlReForClima_read/1 -> Terminator_115/1
  HmiHvacFanLvlReForClima_HmiHvacFanLvlReRiForClima_read/1 -> Terminator_116/1
  HvacFlowRednEnadRe_HvacFlowRednEnadRe_read/1 -> Terminator_117/1
  LowrWtrTActvn_LowrWtrTActvn_read/1 -> Terminator_118/1
  ManModeForRowThrdForModelDial_ManModeForRowThrdForModelDial_read/1 -> Terminator_119/1
  PrkgClimaOnReq_PrkgClimaOnReq_read/1 -> Terminator_120/1
  PrkgClimaOnReqRe_PrkgClimaOnReqRe_read/1 -> Terminator_121/1
  RuranModeSetAirFlowActv_RuranModeSetAirFlowActv_read/1 -> Terminator_122/1
  Ground_1/1 -> ClimaFlowReqForDefrstWithFltMod_ClimaFlowReqForDefrstWithFltMod_write/1
  Ground_2/1 -> ClimaFlowReqForRowFirstAtFlrWithFltMod_ClimaFlowReqForRowFirstAtFlrWithFltMod_write/1
  Ground_3/1 -> ClimaFlowReqForRowFirstAtVentWithFltMod_ClimaFlowReqForRowFirstAtVentWithFltMod_write/1
  Ground_4/1 -> ClimaFlowReqForRowSecAtFlrWithFltMod_ClimaFlowReqForRowSecAtFlrWithFltMod_write/1
  Ground_5/1 -> ClimaFlowReqForRowSecAtVentWithFltMod_ClimaFlowReqForRowSecAtVentWithFltMod_write/1
  Ground_6/1 -> AirFlowAddlForDispCoolg_AirFlowAddlForDispCoolg_write/1
  Ground_7/1 -> ClimaRateVCCAirFlowRampTyp_ClimaRateVCCAirFlowRampTyp_write/1
  Ground_8/1 -> FacForRiLeDifForHvacFlowForVentRowSec_FacForRiLeDifForHvacFlowForVentRowSec_write/1
  Ground_9/1 -> HvacAirMFlowEstimdPredAtStabyForCoolg_HvacAirMFlowEstimdPredAtStabyForCoolg_write/1
  Ground_10/1 -> HvacAirMFlowVentNgcc_HvacAirMFlowVent_write/1
  Ground_11/1 -> HvacFlowFrntReqForDefrst_HvacFlowFrntReqForDefrst_write/1
  Ground_12/1 -> HvacFlowFrntReqForRowFirstAtFlr_HvacFlowFrntReqForRowFirstAtFlr_write/1
  Ground_13/1 -> HvacFlowFrntReqForRowFirstAtFlr_HvacFlowFrntReqForRowFirstAtFlrLe_write/1
  Ground_14/1 -> HvacFlowFrntReqForRowFirstAtFlr_HvacFlowFrntReqForRowFirstAtFlrRi_write/1
  Ground_15/1 -> HvacFlowFrntReqForRowFirstAtVent_HvacFlowFrntReqForRowFirstAtVent_write/1
  Ground_16/1 -> HvacFlowFrntReqForRowFirstAtVent_HvacFlowFrntReqForRowFirstAtVentLe_write/1
  Ground_17/1 -> HvacFlowFrntReqForRowFirstAtVent_HvacFlowFrntReqForRowFirstAtVentLeMid_write/1
  Ground_18/1 -> HvacFlowFrntReqForRowFirstAtVent_HvacFlowFrntReqForRowFirstAtVentLeSide_write/1
  Ground_19/1 -> HvacFlowFrntReqForRowFirstAtVent_HvacFlowFrntReqForRowFirstAtVentRi_write/1
  Ground_20/1 -> HvacFlowFrntReqForRowFirstAtVent_HvacFlowFrntReqForRowFirstAtVentRiMid_write/1
  Ground_21/1 -> HvacFlowFrntReqForRowFirstAtVent_HvacFlowFrntReqForRowFirstAtVentRiSide_write/1
  Ground_22/1 -> HvacFlowFrntReqForRowSecAtFlr_HvacFlowFrntReqForRowSecAtFlr_write/1
  Ground_23/1 -> HvacFlowFrntReqForRowSecAtFlr_HvacFlowFrntReqForRowSecAtFlrLe_write/1
  Ground_24/1 -> HvacFlowFrntReqForRowSecAtFlr_HvacFlowFrntReqForRowSecAtFlrRi_write/1
  Ground_25/1 -> HvacFlowFrntReqForRowSecAtVent_HvacFlowFrntReqForRowSecAtVent_write/1
  Ground_26/1 -> HvacFlowFrntReqForRowSecAtVent_HvacFlowFrntReqForRowSecAtVentLe_write/1
  Ground_27/1 -> HvacFlowFrntReqForRowSecAtVent_HvacFlowFrntReqForRowSecAtVentRi_write/1
  Ground_28/1 -> HvacFlowPredForDefrstAtStaby_HvacFlowPredForDefrstAtStaby_write/1
  Ground_29/1 -> HvacFlowPredForDefrstNoLim_HvacFlowPredForDefrstNoLim_write/1
  Ground_30/1 -> HvacFlowPredForRowFirstAtFlrAtStaby_HvacFlowPredForRowFirstAtFlrAtStaby_write/1
  Ground_31/1 -> HvacFlowPredForRowFirstAtFlrNoLim_HvacFlowPredForRowFirstAtFlrNoLim_write/1
  Ground_32/1 -> HvacFlowPredForRowFirstAtVentAtStaby_HvacFlowPredForRowFirstAtVentAtStaby_write/1
  Ground_33/1 -> HvacFlowPredForRowFirstAtVentNoLim_HvacFlowPredForRowFirstAtVentNoLim_write/1
  Ground_34/1 -> HvacFlowPredForRowSecAtFlrAtStaby_HvacFlowPredForRowSecAtFlrAtStaby_write/1
  Ground_35/1 -> HvacFlowPredForRowSecAtFlrNoLim_HvacFlowPredForRowSecAtFlrNoLim_write/1
  Ground_36/1 -> HvacFlowPredForRowSecAtVentAtStaby_HvacFlowPredForRowSecAtVentAtStaby_write/1
  Ground_37/1 -> HvacFlowPredForRowSecAtVentNoLim_HvacFlowPredForRowSecAtVentNoLim_write/1
  Ground_38/1 -> HvacTotFlowForCmptmt_HvacTotFlowForCmptmt_write/1
  Ground_39/1 -> HvacTotFlowPredAtStaby_HvacTotFlowPredAtStaby_write/1
  Ground_40/1 -> HvacTotFlowPredNoLim_HvacTotFlowPredNoLim_write/1
